simulink model "VirtualADAPT"  (Simulink 8.9)
settings: Solver=ode45  StartTime=0.0  StopTime=inf  RelTol=1e-3  EnableMultiTasking=on
PostLoadFcn:
  | FaultInjectionGUIHandle = FaultInjectionGUI;
InitFcn:
  | VirtualADAPTDataStructure
undefined symbols (not defined in the file or in its folder): ln
libraries: simulink x363
(part 1 of 26)
CloseFcn:
  | close(FaultInjectionGUIHandle);
settings Model: SuppressorTable=22 serialization::archive 11 0 6 0 0 0 0 0  Creator=Indranil Roychoudhury and Matthew Daigle  LastModifiedBy=iroychou
settings OptimizationCC: ExpressionDepthLimit=2147483647  InitFltsAndDblsToZero=on
settings DebuggingCC: ReadBeforeWriteMsg=DisableAll  WriteAfterWriteMsg=DisableAll  WriteAfterReadMsg=DisableAll  SaveWithParameterizedLinksMsg=none  SolverPrmCheckMsg=warning  InheritedTsInSrcMsg=none  UnconnectedInputMsg=none  UnconnectedOutputMsg=none  StrictBusMsg=Warning
settings GRTTargetCC: TargetFcnLib=ansi_tfl_tmw.mat

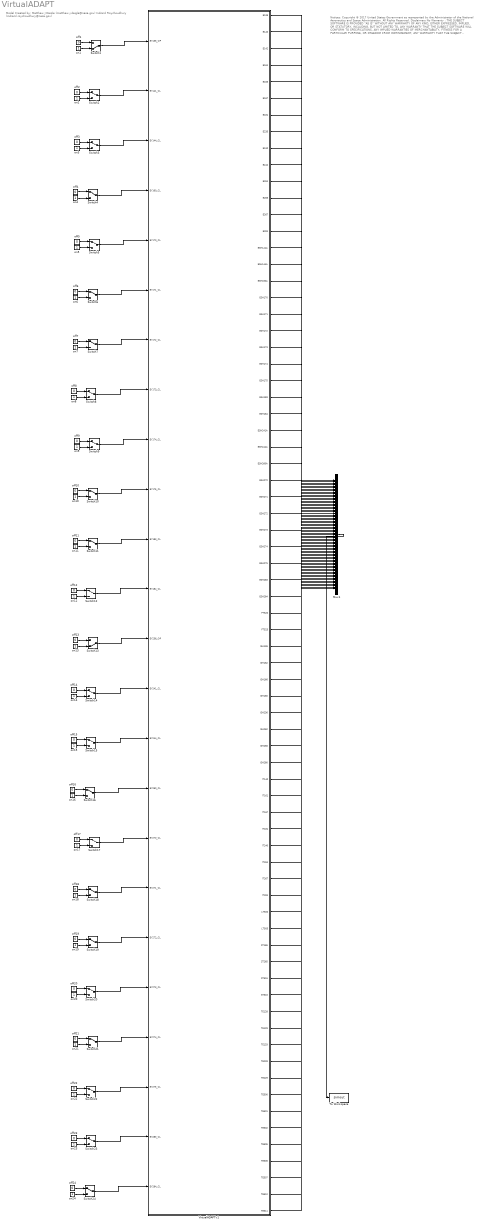
== system VirtualADAPT ==
  note: Notices:
      |
      | Copyright © 2017 United States Government as represented by the Administrator of the National Aeronautics and Space Administration. All Rights Reserved.
      |
      | Disclaimers
      |
      | No Warranty : THE SUBJECT SOFTWARE IS PROVIDED "AS IS" WITHOUT ANY WARRANTY OF ANY KIND, EITHER EXPRESSED, IMPLIED, OR STATUTORY, INCLUDING, BUT NOT LIMITED TO, ANY WARRANTY THAT THE SUBJECT SOFTWARE WILL CONFORM TO SPECIFICATIONS, ANY IMPLIED WARRANTIES OF MERCHANTABILITY, FITNESS FOR A PARTICULAR PURPOSE, OR FREEDOM FROM INFRINGEMENT, ANY WARRANTY THAT THE SUBJECT SOFTWARE WILL BE ERROR FREE, OR ANY WARRANTY THAT DOCUMENTATION, IF PROVIDED, WILL CONFORM TO THE SUBJECT SOFTWARE. THIS AGREEMENT DOES NOT, IN ANY MANNER, CONSTITUTE AN ENDORSEMENT BY GOVERNMENT AGENCY OR ANY PRIOR RECIPIENT OF ANY RESULTS, RESULTING DESIGNS, HARDWARE, SOFTWARE PRODUCTS OR ANY OTHER APPLICATIONS RESULTING FROM USE OF THE SUBJECT SOFTWARE. FURTHER, GOVERNMENT AGENCY DISCLAIMS ALL WARRANTIES AND LIABILITIES REGARDING THIRD-PARTY SOFTWARE, IF PRESENT IN THE ORIGINAL SOFTWARE, AND DISTRIBUTES IT "AS IS."
      |
      | Waiver and Indemnity: RECIPIENT AGREES TO WAIVE ANY AND ALL CLAIMS AGAINST THE UNITED STATES GOVERNMENT, ITS CONTRACTORS AND SUBCONTRACTORS, AS WELL AS ANY PRIOR RECIPIENT. IF RECIPIENT'S USE OF THE SUBJECT SOFTWARE RESULTS IN ANY LIABILITIES, DEMANDS, DAMAGES, EXPENSES OR LOSSES ARISING FROM SUCH USE, INCLUDING ANY DAMAGES FROM PRODUCTS BASED ON, OR RESULTING FROM, RECIPIENT'S USE OF THE SUBJECT SOFTWARE, RECIPIENT SHALL INDEMNIFY AND HOLD HARMLESS THE UNITED STATES GOVERNMENT, ITS CONTRACTORS AND SUBCONTRACTORS, AS WELL AS ANY PRIOR RECIPIENT, TO THE EXTENT PERMITTED BY LAW. RECIPIENT'S SOLE REMEDY FOR ANY SUCH MATTER SHALL BE THE IMMEDIATE, UNILATERAL TERMINATION OF THIS AGREEMENT.
  note: Model Created by:
      | Matthew J Daigle (matthew.j.daigle@nasa.gov)
      | Indranil Roychoudhury (indranil.roychoudhury@nasa.gov)
  Mux1 : Mux  Inputs=73
  Switch1 : ManualSwitch  CurrentSetting=0
  Switch{2..12,14..24} (22 alike) : ManualSwitch
  Switch13 : ManualSwitch  CurrentSetting=0
  To Workspace : ToWorkspace  VariableName=simout  SaveFormat=Structure  SampleTime=-1
  VirtualADAPTv1 : Subsystem
  off{1..24} (24 alike) : Constant  Value=0
  on{1..24} (24 alike) : Constant
  wires:
  off1/1 -> Switch1/1
  on1/1 -> Switch1/2
  off2/1 -> Switch2/1
  on2/1 -> Switch2/2
  off3/1 -> Switch3/1
  on3/1 -> Switch3/2
  off4/1 -> Switch4/1
  on4/1 -> Switch4/2
  off5/1 -> Switch5/1
  on5/1 -> Switch5/2
  off6/1 -> Switch6/1
  on6/1 -> Switch6/2
  off7/1 -> Switch7/1
  on7/1 -> Switch7/2
  off8/1 -> Switch8/1
  on8/1 -> Switch8/2
  off9/1 -> Switch9/1
  on9/1 -> Switch9/2
  off10/1 -> Switch10/1
  on10/1 -> Switch10/2
  off11/1 -> Switch11/1
  on11/1 -> Switch11/2
  off12/1 -> Switch12/1
  on12/1 -> Switch12/2
  off13/1 -> Switch13/1
  on13/1 -> Switch13/2
  off14/1 -> Switch14/1
  on14/1 -> Switch14/2
  off15/1 -> Switch15/1
  on15/1 -> Switch15/2
  off16/1 -> Switch16/1
  on16/1 -> Switch16/2
  off17/1 -> Switch17/1
  on17/1 -> Switch17/2
  off18/1 -> Switch18/1
  on18/1 -> Switch18/2
  off19/1 -> Switch19/1
  on19/1 -> Switch19/2
  off20/1 -> Switch20/1
  on20/1 -> Switch20/2
  off21/1 -> Switch21/1
  on21/1 -> Switch21/2
  off22/1 -> Switch22/1
  on22/1 -> Switch22/2
  off23/1 -> Switch23/1
  on23/1 -> Switch23/2
  off24/1 -> Switch24/1
  on24/1 -> Switch24/2
  Mux1/1 -> To Workspace/1
  Switch1/1 -> VirtualADAPTv1/1(EY136_OP)
  Switch2/1 -> VirtualADAPTv1/2(EY141_CL)
  Switch3/1 -> VirtualADAPTv1/3(EY144_CL)
  Switch4/1 -> VirtualADAPTv1/4(EY160_CL)
  Switch5/1 -> VirtualADAPTv1/5(EY170_CL)
  Switch6/1 -> VirtualADAPTv1/6(EY171_CL)
  Switch7/1 -> VirtualADAPTv1/7(EY172_CL)
  Switch8/1 -> VirtualADAPTv1/8(EY173_CL)
  Switch9/1 -> VirtualADAPTv1/9(EY174_CL)
  Switch10/1 -> VirtualADAPTv1/10(EY175_CL)
  Switch11/1 -> VirtualADAPTv1/11(EY183_CL)
  Switch12/1 -> VirtualADAPTv1/12(EY184_CL)
  Switch13/1 -> VirtualADAPTv1/13(EY236_OP)
  Switch14/1 -> VirtualADAPTv1/14(EY241_CL)
  Switch15/1 -> VirtualADAPTv1/15(EY244_CL)
  Switch16/1 -> VirtualADAPTv1/16(EY260_CL)
  Switch17/1 -> VirtualADAPTv1/17(EY270_CL)
  Switch18/1 -> VirtualADAPTv1/18(EY271_CL)
  Switch19/1 -> VirtualADAPTv1/19(EY272_CL)
  Switch20/1 -> VirtualADAPTv1/20(EY273_CL)
  Switch21/1 -> VirtualADAPTv1/21(EY274_CL)
  Switch22/1 -> VirtualADAPTv1/22(EY275_CL)
  Switch23/1 -> VirtualADAPTv1/23(EY283_CL)
  Switch24/1 -> VirtualADAPTv1/24(EY284_CL)
  VirtualADAPTv1/1(E135) -> Mux1/1
  VirtualADAPTv1/2(E140) -> Mux1/2
  VirtualADAPTv1/3(E142) -> Mux1/3
  VirtualADAPTv1/4(E161) -> Mux1/4
  VirtualADAPTv1/5(E165) -> Mux1/5
  VirtualADAPTv1/6(E167) -> Mux1/6
  VirtualADAPTv1/7(E181) -> Mux1/7
  VirtualADAPTv1/8(E235) -> Mux1/8
  VirtualADAPTv1/9(E240) -> Mux1/9
  VirtualADAPTv1/10(E242) -> Mux1/10
  VirtualADAPTv1/11(E261) -> Mux1/11
  VirtualADAPTv1/12(E265) -> Mux1/12
  VirtualADAPTv1/13(E267) -> Mux1/13
  VirtualADAPTv1/14(E281) -> Mux1/14
  VirtualADAPTv1/15(ESH141A) -> Mux1/15
  VirtualADAPTv1/16(ESH144A) -> Mux1/16
  VirtualADAPTv1/17(ESH160A) -> Mux1/17
  VirtualADAPTv1/18(ESH170) -> Mux1/18
  VirtualADAPTv1/19(ESH171) -> Mux1/19
  VirtualADAPTv1/20(ESH172) -> Mux1/20
  VirtualADAPTv1/21(ESH173) -> Mux1/21
  VirtualADAPTv1/22(ESH174) -> Mux1/22
  VirtualADAPTv1/23(ESH175) -> Mux1/23
  VirtualADAPTv1/24(ESH183) -> Mux1/24
  VirtualADAPTv1/25(ESH184) -> Mux1/25
  VirtualADAPTv1/26(ESH241A) -> Mux1/26
  VirtualADAPTv1/27(ESH244A) -> Mux1/27
  VirtualADAPTv1/28(ESH260A) -> Mux1/28
  VirtualADAPTv1/29(ESH270) -> Mux1/29
  VirtualADAPTv1/30(ESH271) -> Mux1/30
  VirtualADAPTv1/31(ESH272) -> Mux1/31
  VirtualADAPTv1/32(ESH273) -> Mux1/32
  VirtualADAPTv1/33(ESH274) -> Mux1/33
  VirtualADAPTv1/34(ESH275) -> Mux1/34
  VirtualADAPTv1/35(ESH283) -> Mux1/35
  VirtualADAPTv1/36(ESH284) -> Mux1/36
  VirtualADAPTv1/37(FT520) -> Mux1/37
  VirtualADAPTv1/38(FT525) -> Mux1/38
  VirtualADAPTv1/39(ISH136) -> Mux1/39
  VirtualADAPTv1/40(ISH162) -> Mux1/40
  VirtualADAPTv1/41(ISH166) -> Mux1/41
  VirtualADAPTv1/42(ISH180) -> Mux1/42
  VirtualADAPTv1/43(ISH236) -> Mux1/43
  VirtualADAPTv1/44(ISH262) -> Mux1/44
  VirtualADAPTv1/45(ISH266) -> Mux1/45
  VirtualADAPTv1/46(ISH280) -> Mux1/46
  VirtualADAPTv1/47(IT140) -> Mux1/47
  VirtualADAPTv1/48(IT161) -> Mux1/48
  VirtualADAPTv1/49(IT167) -> Mux1/49
  VirtualADAPTv1/50(IT181) -> Mux1/50
  VirtualADAPTv1/51(IT240) -> Mux1/51
  VirtualADAPTv1/52(IT261) -> Mux1/52
  VirtualADAPTv1/53(IT267) -> Mux1/53
  VirtualADAPTv1/54(IT281) -> Mux1/54
  VirtualADAPTv1/55(LT500) -> Mux1/55
  VirtualADAPTv1/56(LT505) -> Mux1/56
  VirtualADAPTv1/57(ST165) -> Mux1/57
  VirtualADAPTv1/58(ST265) -> Mux1/58
  VirtualADAPTv1/59(ST515) -> Mux1/59
  VirtualADAPTv1/60(ST516) -> Mux1/60
  VirtualADAPTv1/61(TE128) -> Mux1/61
  VirtualADAPTv1/62(TE129) -> Mux1/62
  VirtualADAPTv1/63(TE133) -> Mux1/63
  VirtualADAPTv1/64(TE228) -> Mux1/64
  VirtualADAPTv1/65(TE229) -> Mux1/65
  VirtualADAPTv1/66(TE500) -> Mux1/66
  VirtualADAPTv1/67(TE501) -> Mux1/67
  VirtualADAPTv1/68(TE502) -> Mux1/68
  VirtualADAPTv1/69(TE505) -> Mux1/69
  VirtualADAPTv1/70(TE506) -> Mux1/70
  VirtualADAPTv1/71(TE507) -> Mux1/71
  VirtualADAPTv1/72(TE510) -> Mux1/72
  VirtualADAPTv1/73(TE511) -> Mux1/73

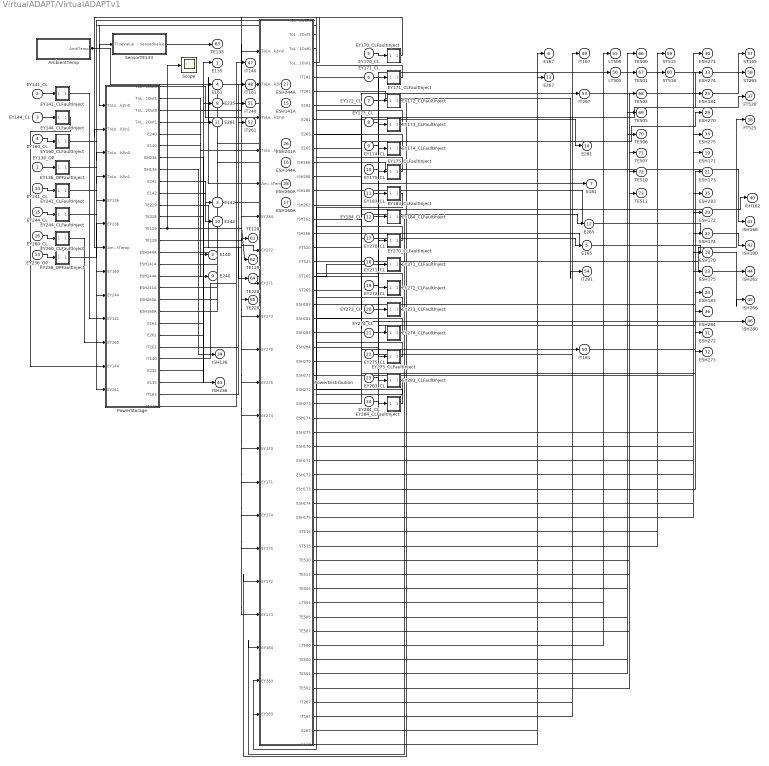
== system VirtualADAPT/VirtualADAPTv1 ==
  EY136_OP : Inport
  EY141_CL : Inport  Port=2
  EY144_CL : Inport  Port=3
  EY160_CL : Inport  Port=4
  EY170_CL : Inport  Port=5
  EY171_CL : Inport  Port=6
  EY172_CL : Inport  Port=7
  EY173_CL : Inport  Port=8
  EY174_CL : Inport  Port=9
  EY175_CL : Inport  Port=10
  EY183_CL : Inport  Port=11
  EY184_CL : Inport  Port=12
  EY236_OP : Inport  Port=13
  EY241_CL : Inport  Port=14
  EY244_CL : Inport  Port=15
  EY260_CL : Inport  Port=16
  EY270_CL : Inport  Port=17
  EY271_CL : Inport  Port=18
  EY272_CL : Inport  Port=19
  EY273_CL : Inport  Port=20
  EY274_CL : Inport  Port=21
  EY275_CL : Inport  Port=22
  EY283_CL : Inport  Port=23
  EY284_CL : Inport  Port=24
  AmbientTemp : Subsystem
  EY136_OPFaultInject : Subsystem  mask{FP=Nominal, FT=0, FM=1}
      mask prompts: FP "Fault Profile:"; FT "Fault Injection Time:"; FM "Stuck Value"
      mask TypeOptions FP: Nominal | StuckAt
      mask Display: image(imread('FaultInject.png','BackgroundColor',[1 1 1]))
  EY141_CLFaultInject : Subsystem  mask{FP=Nominal, FT=0, FM=0}
      mask prompts: FP "Fault Profile:"; FT "Fault Injection Time:"; FM "Stuck Value"
      mask TypeOptions FP: Nominal | StuckAt
      mask Display: image(imread('FaultInject.png','BackgroundColor',[1 1 1]))
  EY144_CLFaultInject : Subsystem  mask{FP=Nominal, FT=0, FM=0}
      mask prompts: FP "Fault Profile:"; FT "Fault Injection Time:"; FM "Stuck Value"
      mask TypeOptions FP: Nominal | StuckAt
      mask Display: image(imread('FaultInject.png','BackgroundColor',[1 1 1]))
  EY160_CLFaultInject : Subsystem  mask{FP=Nominal, FT=0, FM=0}
      mask prompts: FP "Fault Profile:"; FT "Fault Injection Time:"; FM "Stuck Value"
      mask TypeOptions FP: Nominal | StuckAt
      mask Display: image(imread('FaultInject.png','BackgroundColor',[1 1 1]))
  EY170_CLFaultInject : Subsystem  mask{FP=Nominal, FT=0, FM=0}
      mask prompts: FP "Fault Profile:"; FT "Fault Injection Time:"; FM "Stuck Value"
      mask TypeOptions FP: Nominal | StuckAt
      mask Display: image(imread('FaultInject.png','BackgroundColor',[1 1 1]))
  EY171_CLFaultInject : Subsystem  mask{FP=Nominal, FT=0, FM=0}
      mask prompts: FP "Fault Profile:"; FT "Fault Injection Time:"; FM "Stuck Value"
      mask TypeOptions FP: Nominal | StuckAt
      mask Display: image(imread('FaultInject.png','BackgroundColor',[1 1 1]))
  EY172_CLFaultInject : Subsystem  mask{FP=Nominal, FT=0, FM=0}
      mask prompts: FP "Fault Profile:"; FT "Fault Injection Time:"; FM "Stuck Value"
      mask TypeOptions FP: Nominal | StuckAt
      mask Display: image(imread('FaultInject.png','BackgroundColor',[1 1 1]))
  EY173_CLFaultInject : Subsystem  mask{FP=Nominal, FT=0, FM=0}
      mask prompts: FP "Fault Profile:"; FT "Fault Injection Time:"; FM "Stuck Value"
      mask TypeOptions FP: Nominal | StuckAt
      mask Display: image(imread('FaultInject.png','BackgroundColor',[1 1 1]))
  EY174_CLFaultInject : Subsystem  mask{FP=Nominal, FT=0, FM=0}
      mask prompts: FP "Fault Profile:"; FT "Fault Injection Time:"; FM "Stuck Value"
      mask TypeOptions FP: Nominal | StuckAt
      mask Display: image(imread('FaultInject.png','BackgroundColor',[1 1 1]))
  EY175_CLFaultInject : Subsystem  mask{FP=Nominal, FT=0, FM=0}
      mask prompts: FP "Fault Profile:"; FT "Fault Injection Time:"; FM "Stuck Value"
      mask TypeOptions FP: Nominal | StuckAt
      mask Display: image(imread('FaultInject.png','BackgroundColor',[1 1 1]))
  EY183_CLFaultInject : Subsystem  mask{FP=Nominal, FT=0, FM=0}
      mask prompts: FP "Fault Profile:"; FT "Fault Injection Time:"; FM "Stuck Value"
      mask TypeOptions FP: Nominal | StuckAt
      mask Display: image(imread('FaultInject.png','BackgroundColor',[1 1 1]))
  EY184_CLFaultInject : Subsystem  mask{FP=Nominal, FT=0, FM=0}
      mask prompts: FP "Fault Profile:"; FT "Fault Injection Time:"; FM "Stuck Value"
      mask TypeOptions FP: Nominal | StuckAt
      mask Display: image(imread('FaultInject.png','BackgroundColor',[1 1 1]))
  EY236_OPFaultInject : Subsystem  mask{FP=Nominal, FT=0, FM=0}
      mask prompts: FP "Fault Profile:"; FT "Fault Injection Time:"; FM "Stuck Value"
      mask TypeOptions FP: Nominal | StuckAt
      mask Display: image(imread('FaultInject.png','BackgroundColor',[1 1 1]))
  EY241_CLFaultInject : Subsystem  mask{FP=Nominal, FT=0, FM=0}
      mask prompts: FP "Fault Profile:"; FT "Fault Injection Time:"; FM "Stuck Value"
      mask TypeOptions FP: Nominal | StuckAt
      mask Display: image(imread('FaultInject.png','BackgroundColor',[1 1 1]))
  EY244_CLFaultInject : Subsystem  mask{FP=Nominal, FT=0, FM=0}
      mask prompts: FP "Fault Profile:"; FT "Fault Injection Time:"; FM "Stuck Value"
      mask TypeOptions FP: Nominal | StuckAt
      mask Display: image(imread('FaultInject.png','BackgroundColor',[1 1 1]))
  EY260_CLFaultInject : Subsystem  mask{FP=Nominal, FT=0, FM=0}
      mask prompts: FP "Fault Profile:"; FT "Fault Injection Time:"; FM "Stuck Value"
      mask TypeOptions FP: Nominal | StuckAt
      mask Display: image(imread('FaultInject.png','BackgroundColor',[1 1 1]))
  EY270_CLFaultInject : Subsystem  mask{FP=Nominal, FT=0, FM=0}
      mask prompts: FP "Fault Profile:"; FT "Fault Injection Time:"; FM "Stuck Value"
      mask TypeOptions FP: Nominal | StuckAt
      mask Display: image(imread('FaultInject.png','BackgroundColor',[1 1 1]))
  EY271_CLFaultInject : Subsystem  mask{FP=Nominal, FT=0, FM=0}
      mask prompts: FP "Fault Profile:"; FT "Fault Injection Time:"; FM "Stuck Value"
      mask TypeOptions FP: Nominal | StuckAt
      mask Display: image(imread('FaultInject.png','BackgroundColor',[1 1 1]))
  EY272_CLFaultInject : Subsystem  mask{FP=Nominal, FT=0, FM=0}
      mask prompts: FP "Fault Profile:"; FT "Fault Injection Time:"; FM "Stuck Value"
      mask TypeOptions FP: Nominal | StuckAt
      mask Display: image(imread('FaultInject.png','BackgroundColor',[1 1 1]))
  EY273_CLFaultInject : Subsystem  mask{FP=Nominal, FT=0, FM=0}
      mask prompts: FP "Fault Profile:"; FT "Fault Injection Time:"; FM "Stuck Value"
      mask TypeOptions FP: Nominal | StuckAt
      mask Display: image(imread('FaultInject.png','BackgroundColor',[1 1 1]))
  EY274_CLFaultInject : Subsystem  mask{FP=Nominal, FT=0, FM=0}
      mask prompts: FP "Fault Profile:"; FT "Fault Injection Time:"; FM "Stuck Value"
      mask TypeOptions FP: Nominal | StuckAt
      mask Display: image(imread('FaultInject.png','BackgroundColor',[1 1 1]))
  EY275_CLFaultInject : Subsystem  mask{FP=Nominal, FT=0, FM=0}
      mask prompts: FP "Fault Profile:"; FT "Fault Injection Time:"; FM "Stuck Value"
      mask TypeOptions FP: Nominal | StuckAt
      mask Display: image(imread('FaultInject.png','BackgroundColor',[1 1 1]))
  EY283_CLFaultInject : Subsystem  mask{FP=Nominal, FT=0, FM=0}
      mask prompts: FP "Fault Profile:"; FT "Fault Injection Time:"; FM "Stuck Value"
      mask TypeOptions FP: Nominal | StuckAt
      mask Display: image(imread('FaultInject.png','BackgroundColor',[1 1 1]))
  EY284_CLFaultInject : Subsystem  mask{FP=Nominal, FT=0, FM=0}
      mask prompts: FP "Fault Profile:"; FT "Fault Injection Time:"; FM "Stuck Value"
      mask TypeOptions FP: Nominal | StuckAt
      mask Display: image(imread('FaultInject.png','BackgroundColor',[1 1 1]))
  PowerDistribution : Subsystem
  PowerStorage : Subsystem
  Scope : Scope
  SensorTE133 : Subsystem
  E135 : Outport
  E140 : Outport  Port=2
  E142 : Outport  Port=3
  E161 : Outport  Port=4
  E165 : Outport  Port=5
  E167 : Outport  Port=6
  E181 : Outport  Port=7
  E235 : Outport  Port=8
  E240 : Outport  Port=9
  E242 : Outport  Port=10
  E261 : Outport  Port=11
  E265 : Outport  Port=12
  E267 : Outport  Port=13
  E281 : Outport  Port=14
  ESH141A : Outport  Port=15
  ESH144A : Outport  Port=16
  ESH160A : Outport  Port=17
  ESH170 : Outport  Port=18
  ESH171 : Outport  Port=19
  ESH172 : Outport  Port=20
  ESH173 : Outport  Port=21
  ESH174 : Outport  Port=22
  ESH175 : Outport  Port=23
  ESH183 : Outport  Port=24
  ESH184 : Outport  Port=25
  ESH241A : Outport  Port=26
  ESH244A : Outport  Port=27
  ESH260A : Outport  Port=28
  ESH270 : Outport  Port=29
  ESH271 : Outport  Port=30
  ESH272 : Outport  Port=31
  ESH273 : Outport  Port=32
  ESH274 : Outport  Port=33
  ESH275 : Outport  Port=34
  ESH283 : Outport  Port=35
  ESH284 : Outport  Port=36
  FT520 : Outport  Port=37
  FT525 : Outport  Port=38
  ISH136 : Outport  Port=39
  ISH162 : Outport  Port=40
  ISH166 : Outport  Port=41
  ISH180 : Outport  Port=42
  ISH236 : Outport  Port=43
  ISH262 : Outport  Port=44
  ISH266 : Outport  Port=45
  ISH280 : Outport  Port=46
  IT140 : Outport  Port=47
  IT161 : Outport  Port=48
  IT167 : Outport  Port=49
  IT181 : Outport  Port=50
  IT240 : Outport  Port=51
  IT261 : Outport  Port=52
  IT267 : Outport  Port=53
  IT281 : Outport  Port=54
  LT500 : Outport  Port=55
  LT505 : Outport  Port=56
  ST165 : Outport  Port=57
  ST265 : Outport  Port=58
  ST515 : Outport  Port=59
  ST516 : Outport  Port=60
  TE128 : Outport  Port=61
  TE129 : Outport  Port=62
  TE133 : Outport  Port=63
  TE228 : Outport  Port=64
  TE229 : Outport  Port=65
  TE500 : Outport  Port=66
  TE501 : Outport  Port=67
  TE502 : Outport  Port=68
  TE505 : Outport  Port=69
  TE506 : Outport  Port=70
  TE507 : Outport  Port=71
  TE510 : Outport  Port=72
  TE511 : Outport  Port=73
  wires:
  EY271_CL/1 -> EY271_CLFaultInject/1(In)
  EY270_CL/1 -> EY270_CLFaultInject/1(In)
  EY275_CL/1 -> EY275_CLFaultInject/1(In)
  EY174_CL/1 -> EY174_CLFaultInject/1(In)
  EY170_CL/1 -> EY170_CLFaultInject/1(In)
  EY273_CL/1 -> EY273_CLFaultInject/1(In)
  EY175_CL/1 -> EY175_CLFaultInject/1(In)
  EY171_CL/1 -> EY171_CLFaultInject/1(In)
  EY172_CL/1 -> EY172_CLFaultInject/1(In)
  EY272_CL/1 -> EY272_CLFaultInject/1(In)
  EY283_CL/1 -> EY283_CLFaultInject/1(In)
  EY173_CL/1 -> EY173_CLFaultInject/1(In)
  EY184_CL/1 -> EY184_CLFaultInject/1(In)
  EY183_CL/1 -> EY183_CLFaultInject/1(In)
  EY260_CL/1 -> EY260_CLFaultInject/1(In)
  EY141_CL/1 -> EY141_CLFaultInject/1(In)
  EY144_CL/1 -> EY144_CLFaultInject/1(In)
  EY160_CL/1 -> EY160_CLFaultInject/1(In)
  EY244_CL/1 -> EY244_CLFaultInject/1(In)
  EY241_CL/1 -> EY241_CLFaultInject/1(In)
  EY136_OP/1 -> EY136_OPFaultInject/1(In)
  EY284_CL/1 -> EY284_CLFaultInject/1(In)
  EY274_CL/1 -> EY274_CLFaultInject/1(In)
  EY236_OP/1 -> EY236_OPFaultInject/1(In)
  PowerStorage/1(ToLoadBank1Out0) -> PowerDistribution/3(ToLoadBank1In0)
  PowerDistribution/3(ToLoadBank1Out0) -> PowerStorage/1(ToLoadBank1In0)
  PowerStorage/2(ToLoadBank1Out1) -> PowerDistribution/4(ToLoadBank1In1)
  PowerDistribution/4(ToLoadBank1Out1) -> PowerStorage/2(ToLoadBank1In1)
  PowerStorage/3(ToLoadBank2Out0) -> PowerDistribution/1(ToLoadBank2In0)
  PowerDistribution/1(ToLoadBank2Out0) -> PowerStorage/3(ToLoadBank2In0)
  PowerStorage/4(ToLoadBank2Out1) -> PowerDistribution/2(ToLoadBank2In1)
  PowerDistribution/2(ToLoadBank2Out1) -> PowerStorage/4(ToLoadBank2In1)
  AmbientTemp/1(AmbTemp) -> PowerDistribution/5(AmbientTemp), SensorTE133/1(TrueValue), PowerStorage/7(AmbientTemp)
  EY283_CLFaultInject/1(Out) -> PowerDistribution/20(EY283)
  EY184_CLFaultInject/1(Out) -> PowerDistribution/19(EY184)
  EY170_CLFaultInject/1(Out) -> PowerDistribution/13(EY170)
  EY260_CLFaultInject/1(Out) -> PowerStorage/11(EY260)
  EY273_CLFaultInject/1(Out) -> PowerDistribution/9(EY273)
  EY271_CLFaultInject/1(Out) -> PowerDistribution/8(EY271)
  EY144_CLFaultInject/1(Out) -> PowerStorage/12(EY144)
  EY141_CLFaultInject/1(Out) -> PowerStorage/10(EY141)
  EY136_OPFaultInject/1(Out) -> PowerStorage/5(EY136)
  EY275_CLFaultInject/1(Out) -> PowerDistribution/11(EY275)
  EY284_CLFaultInject/1(Out) -> PowerDistribution/6(EY284)
  EY160_CLFaultInject/1(Out) -> PowerStorage/8(EY160)
  EY244_CLFaultInject/1(Out) -> PowerStorage/9(EY244)
  EY236_OPFaultInject/1(Out) -> PowerStorage/6(EY236)
  EY241_CLFaultInject/1(Out) -> PowerStorage/13(EY241)
  EY172_CLFaultInject/1(Out) -> PowerDistribution/17(EY172)
  EY270_CLFaultInject/1(Out) -> PowerDistribution/10(EY270)
  EY274_CLFaultInject/1(Out) -> PowerDistribution/12(EY274)
  EY175_CLFaultInject/1(Out) -> PowerDistribution/16(EY175)
  EY174_CLFaultInject/1(Out) -> PowerDistribution/15(EY174)
  EY173_CLFaultInject/1(Out) -> PowerDistribution/18(EY173)
  EY272_CLFaultInject/1(Out) -> PowerDistribution/7(EY272)
  EY183_CLFaultInject/1(Out) -> PowerDistribution/21(EY183)
  EY171_CLFaultInject/1(Out) -> PowerDistribution/14(EY171)
  PowerDistribution/34(ESH173) -> ESH173/1
  PowerDistribution/27(ESH272) -> ESH272/1
  PowerDistribution/35(ESH174) -> ESH174/1
  PowerDistribution/36(ESH175) -> ESH175/1
  PowerDistribution/24(ESH284) -> ESH284/1
  PowerStorage/21(E161) -> E161/1
  PowerStorage/26(E135) -> E135/1
  PowerStorage/25(E235) -> E235/1
  PowerStorage/22(E261) -> E261/1
  PowerDistribution/11(ISH166) -> ISH166/1
  PowerStorage/27(IT161) -> IT161/1
  PowerStorage/23(IT261) -> IT261/1
  PowerDistribution/48(TE502) -> TE502/1
  PowerDistribution/14(ISH262) -> ISH262/1
  PowerDistribution/45(LT500) -> LT500/1
  PowerStorage/11(TE229) -> TE229/1
  PowerDistribution/13(ISH180) -> ISH180/1
  PowerDistribution/12(ISH280) -> ISH280/1
  PowerDistribution/16(ISH266) -> ISH266/1
  PowerDistribution/10(E165) -> E165/1
  PowerDistribution/50(IT167) -> IT167/1
  PowerDistribution/25(ESH270) -> ESH270/1
  PowerDistribution/17(FT520) -> FT520/1
  PowerDistribution/20(ST265) -> ST265/1
  PowerStorage/16(ESH141A) -> ESH141A/1
  PowerDistribution/30(ESH275) -> ESH275/1
  PowerDistribution/15(ISH162) -> ISH162/1
  PowerDistribution/52(E167) -> E167/1
  PowerDistribution/42(LT505) -> LT505/1
  SensorTE133/1(SensedValue) -> TE133/1
  PowerDistribution/8(E281) -> E281/1
  PowerStorage/18(ESH241A) -> ESH241A/1
  PowerStorage/7(ISH236) -> ISH236/1
  PowerDistribution/51(E267) -> E267/1
  PowerStorage/15(ESH144A) -> ESH144A/1
  PowerStorage/5(E240) -> E240/1
  PowerDistribution/47(TE501) -> TE501/1
  PowerDistribution/22(ESH184) -> ESH184/1
  PowerDistribution/18(FT525) -> FT525/1
  PowerDistribution/38(ST515) -> ST515/1
  PowerDistribution/28(ESH273) -> ESH273/1
  PowerDistribution/21(ESH183) -> ESH183/1
  PowerDistribution/44(TE507) -> TE507/1
  PowerDistribution/46(TE500) -> TE500/1
  PowerDistribution/29(ESH274) -> ESH274/1
  PowerDistribution/23(ESH283) -> ESH283/1
  PowerDistribution/39(TE510) -> TE510/1
  PowerDistribution/32(ESH171) -> ESH171/1
  PowerStorage/17(ESH244A) -> ESH244A/1
  PowerDistribution/41(TE505) -> TE505/1
  PowerStorage/13(TE129) -> TE129/1, Scope/1
  PowerStorage/14(TE128) -> TE128/1
  PowerDistribution/26(ESH271) -> ESH271/1
  PowerDistribution/33(ESH172) -> ESH172/1
  PowerDistribution/7(E181) -> E181/1
  PowerDistribution/43(TE506) -> TE506/1
  PowerStorage/20(ESH160A) -> ESH160A/1
  PowerStorage/6(E140) -> E140/1
  PowerStorage/9(E242) -> E242/1
  PowerStorage/12(TE228) -> TE228/1
  PowerStorage/10(E142) -> E142/1
  PowerStorage/19(ESH260A) -> ESH260A/1
  PowerStorage/24(IT140) -> IT140/1
  PowerDistribution/9(E265) -> E265/1
  PowerDistribution/49(IT267) -> IT267/1
  PowerDistribution/6(IT281) -> IT281/1
  PowerDistribution/40(TE511) -> TE511/1
  PowerStorage/8(ISH136) -> ISH136/1
  PowerStorage/28(IT240) -> IT240/1
  PowerDistribution/37(ST516) -> ST516/1
  PowerDistribution/31(ESH170) -> ESH170/1
  PowerDistribution/19(ST165) -> ST165/1
  PowerDistribution/5(IT181) -> IT181/1

== system VirtualADAPT/VirtualADAPTv1/AmbientTemp ==
  AmbientTemp : Subsystem  mask{Constant=22, FP=Nominal, FT=0, FM=0}
      mask prompts: Constant "Value of Constant:"; FP "Fault Profile:"; FT "Fault Injection Time:"; FM "Fault Magnitude/Slope"
      mask TypeOptions FP: Nominal | Abrupt | Incipient
      mask Display: image(imread('Constant.png','BackgroundColor',[1 1 1]))
  AmbTemp : Outport
  wires:
  AmbientTemp/1(C) -> AmbTemp/1

== system VirtualADAPT/VirtualADAPTv1/EY136_OPFaultInject ==
  In : Inport
  Fault Inject : Subsystem
  Fault Profile : Step  Time=FT  Before=1  After=FP+(FP-1)*3  SampleTime=0
  Filler : Constant  Value=0
  StuckAt : Constant  Value=FM
  Out : Outport
  wires:
  Fault Profile/1 -> Fault Inject/2(Fault Profile)
  Filler/1 -> Fault Inject/3(Step), Fault Inject/4(Ramp), Fault Inject/5(Bias)
  In/1 -> Fault Inject/1(Nominal)
  Fault Inject/1(Out1) -> Out/1
  StuckAt/1 -> Fault Inject/6(stuckAt)

== system VirtualADAPT/VirtualADAPTv1/EY141_CLFaultInject ==
  In : Inport
  Fault Inject : Subsystem
  Fault Profile : Step  Time=FT  Before=1  After=FP+(FP-1)*3  SampleTime=0
  Filler : Constant  Value=0
  StuckAt : Constant  Value=FM
  Out : Outport
  wires:
  Fault Profile/1 -> Fault Inject/2(Fault Profile)
  Filler/1 -> Fault Inject/3(Step), Fault Inject/4(Ramp), Fault Inject/5(Bias)
  In/1 -> Fault Inject/1(Nominal)
  Fault Inject/1(Out1) -> Out/1
  StuckAt/1 -> Fault Inject/6(stuckAt)

== system VirtualADAPT/VirtualADAPTv1/EY144_CLFaultInject ==
  In : Inport
  Fault Inject : Subsystem
  Fault Profile : Step  Time=FT  Before=1  After=FP+(FP-1)*3  SampleTime=0
  Filler : Constant  Value=0
  StuckAt : Constant  Value=FM
  Out : Outport
  wires:
  Fault Profile/1 -> Fault Inject/2(Fault Profile)
  Filler/1 -> Fault Inject/3(Step), Fault Inject/4(Ramp), Fault Inject/5(Bias)
  In/1 -> Fault Inject/1(Nominal)
  Fault Inject/1(Out1) -> Out/1
  StuckAt/1 -> Fault Inject/6(stuckAt)

== system VirtualADAPT/VirtualADAPTv1/EY160_CLFaultInject ==
  In : Inport
  Fault Inject : Subsystem
  Fault Profile : Step  Time=FT  Before=1  After=FP+(FP-1)*3  SampleTime=0
  Filler : Constant  Value=0
  StuckAt : Constant  Value=FM
  Out : Outport
  wires:
  Fault Profile/1 -> Fault Inject/2(Fault Profile)
  Filler/1 -> Fault Inject/3(Step), Fault Inject/4(Ramp), Fault Inject/5(Bias)
  In/1 -> Fault Inject/1(Nominal)
  Fault Inject/1(Out1) -> Out/1
  StuckAt/1 -> Fault Inject/6(stuckAt)

== system VirtualADAPT/VirtualADAPTv1/EY170_CLFaultInject ==
  In : Inport
  Fault Inject : Subsystem
  Fault Profile : Step  Time=FT  Before=1  After=FP+(FP-1)*3  SampleTime=0
  Filler : Constant  Value=0
  StuckAt : Constant  Value=FM
  Out : Outport
  wires:
  Fault Profile/1 -> Fault Inject/2(Fault Profile)
  Filler/1 -> Fault Inject/3(Step), Fault Inject/4(Ramp), Fault Inject/5(Bias)
  In/1 -> Fault Inject/1(Nominal)
  Fault Inject/1(Out1) -> Out/1
  StuckAt/1 -> Fault Inject/6(stuckAt)

== system VirtualADAPT/VirtualADAPTv1/EY171_CLFaultInject ==
  In : Inport
  Fault Inject : Subsystem
  Fault Profile : Step  Time=FT  Before=1  After=FP+(FP-1)*3  SampleTime=0
  Filler : Constant  Value=0
  StuckAt : Constant  Value=FM
  Out : Outport
  wires:
  Fault Profile/1 -> Fault Inject/2(Fault Profile)
  Filler/1 -> Fault Inject/3(Step), Fault Inject/4(Ramp), Fault Inject/5(Bias)
  In/1 -> Fault Inject/1(Nominal)
  Fault Inject/1(Out1) -> Out/1
  StuckAt/1 -> Fault Inject/6(stuckAt)

== system VirtualADAPT/VirtualADAPTv1/EY172_CLFaultInject ==
  In : Inport
  Fault Inject : Subsystem
  Fault Profile : Step  Time=FT  Before=1  After=FP+(FP-1)*3  SampleTime=0
  Filler : Constant  Value=0
  StuckAt : Constant  Value=FM
  Out : Outport
  wires:
  Fault Profile/1 -> Fault Inject/2(Fault Profile)
  Filler/1 -> Fault Inject/3(Step), Fault Inject/4(Ramp), Fault Inject/5(Bias)
  In/1 -> Fault Inject/1(Nominal)
  Fault Inject/1(Out1) -> Out/1
  StuckAt/1 -> Fault Inject/6(stuckAt)

== system VirtualADAPT/VirtualADAPTv1/EY173_CLFaultInject ==
  In : Inport
  Fault Inject : Subsystem
  Fault Profile : Step  Time=FT  Before=1  After=FP+(FP-1)*3  SampleTime=0
  Filler : Constant  Value=0
  StuckAt : Constant  Value=FM
  Out : Outport
  wires:
  Fault Profile/1 -> Fault Inject/2(Fault Profile)
  Filler/1 -> Fault Inject/3(Step), Fault Inject/4(Ramp), Fault Inject/5(Bias)
  In/1 -> Fault Inject/1(Nominal)
  Fault Inject/1(Out1) -> Out/1
  StuckAt/1 -> Fault Inject/6(stuckAt)

== system VirtualADAPT/VirtualADAPTv1/EY174_CLFaultInject ==
  In : Inport
  Fault Inject : Subsystem
  Fault Profile : Step  Time=FT  Before=1  After=FP+(FP-1)*3  SampleTime=0
  Filler : Constant  Value=0
  StuckAt : Constant  Value=FM
  Out : Outport
  wires:
  Fault Profile/1 -> Fault Inject/2(Fault Profile)
  Filler/1 -> Fault Inject/3(Step), Fault Inject/4(Ramp), Fault Inject/5(Bias)
  In/1 -> Fault Inject/1(Nominal)
  Fault Inject/1(Out1) -> Out/1
  StuckAt/1 -> Fault Inject/6(stuckAt)

== system VirtualADAPT/VirtualADAPTv1/EY175_CLFaultInject ==
  In : Inport
  Fault Inject : Subsystem
  Fault Profile : Step  Time=FT  Before=1  After=FP+(FP-1)*3  SampleTime=0
  Filler : Constant  Value=0
  StuckAt : Constant  Value=FM
  Out : Outport
  wires:
  Fault Profile/1 -> Fault Inject/2(Fault Profile)
  Filler/1 -> Fault Inject/3(Step), Fault Inject/4(Ramp), Fault Inject/5(Bias)
  In/1 -> Fault Inject/1(Nominal)
  Fault Inject/1(Out1) -> Out/1
  StuckAt/1 -> Fault Inject/6(stuckAt)

== system VirtualADAPT/VirtualADAPTv1/EY183_CLFaultInject ==
  In : Inport
  Fault Inject : Subsystem
  Fault Profile : Step  Time=FT  Before=1  After=FP+(FP-1)*3  SampleTime=0
  Filler : Constant  Value=0
  StuckAt : Constant  Value=FM
  Out : Outport
  wires:
  Fault Profile/1 -> Fault Inject/2(Fault Profile)
  Filler/1 -> Fault Inject/3(Step), Fault Inject/4(Ramp), Fault Inject/5(Bias)
  In/1 -> Fault Inject/1(Nominal)
  Fault Inject/1(Out1) -> Out/1
  StuckAt/1 -> Fault Inject/6(stuckAt)

== system VirtualADAPT/VirtualADAPTv1/EY184_CLFaultInject ==
  In : Inport
  Fault Inject : Subsystem
  Fault Profile : Step  Time=FT  Before=1  After=FP+(FP-1)*3  SampleTime=0
  Filler : Constant  Value=0
  StuckAt : Constant  Value=FM
  Out : Outport
  wires:
  Fault Profile/1 -> Fault Inject/2(Fault Profile)
  Filler/1 -> Fault Inject/3(Step), Fault Inject/4(Ramp), Fault Inject/5(Bias)
  In/1 -> Fault Inject/1(Nominal)
  Fault Inject/1(Out1) -> Out/1
  StuckAt/1 -> Fault Inject/6(stuckAt)

== system VirtualADAPT/VirtualADAPTv1/EY236_OPFaultInject ==
  In : Inport
  Fault Inject : Subsystem
  Fault Profile : Step  Time=FT  Before=1  After=FP+(FP-1)*3  SampleTime=0
  Filler : Constant  Value=0
  StuckAt : Constant  Value=FM
  Out : Outport
  wires:
  Fault Profile/1 -> Fault Inject/2(Fault Profile)
  Filler/1 -> Fault Inject/3(Step), Fault Inject/4(Ramp), Fault Inject/5(Bias)
  In/1 -> Fault Inject/1(Nominal)
  Fault Inject/1(Out1) -> Out/1
  StuckAt/1 -> Fault Inject/6(stuckAt)

== system VirtualADAPT/VirtualADAPTv1/EY241_CLFaultInject ==
  In : Inport
  Fault Inject : Subsystem
  Fault Profile : Step  Time=FT  Before=1  After=FP+(FP-1)*3  SampleTime=0
  Filler : Constant  Value=0
  StuckAt : Constant  Value=FM
  Out : Outport
  wires:
  Fault Profile/1 -> Fault Inject/2(Fault Profile)
  Filler/1 -> Fault Inject/3(Step), Fault Inject/4(Ramp), Fault Inject/5(Bias)
  In/1 -> Fault Inject/1(Nominal)
  Fault Inject/1(Out1) -> Out/1
  StuckAt/1 -> Fault Inject/6(stuckAt)

== system VirtualADAPT/VirtualADAPTv1/EY244_CLFaultInject ==
  In : Inport
  Fault Inject : Subsystem
  Fault Profile : Step  Time=FT  Before=1  After=FP+(FP-1)*3  SampleTime=0
  Filler : Constant  Value=0
  StuckAt : Constant  Value=FM
  Out : Outport
  wires:
  Fault Profile/1 -> Fault Inject/2(Fault Profile)
  Filler/1 -> Fault Inject/3(Step), Fault Inject/4(Ramp), Fault Inject/5(Bias)
  In/1 -> Fault Inject/1(Nominal)
  Fault Inject/1(Out1) -> Out/1
  StuckAt/1 -> Fault Inject/6(stuckAt)

== system VirtualADAPT/VirtualADAPTv1/EY260_CLFaultInject ==
  In : Inport
  Fault Inject : Subsystem
  Fault Profile : Step  Time=FT  Before=1  After=FP+(FP-1)*3  SampleTime=0
  Filler : Constant  Value=0
  StuckAt : Constant  Value=FM
  Out : Outport
  wires:
  Fault Profile/1 -> Fault Inject/2(Fault Profile)
  Filler/1 -> Fault Inject/3(Step), Fault Inject/4(Ramp), Fault Inject/5(Bias)
  In/1 -> Fault Inject/1(Nominal)
  Fault Inject/1(Out1) -> Out/1
  StuckAt/1 -> Fault Inject/6(stuckAt)

== system VirtualADAPT/VirtualADAPTv1/EY270_CLFaultInject ==
  In : Inport
  Fault Inject : Subsystem
  Fault Profile : Step  Time=FT  Before=1  After=FP+(FP-1)*3  SampleTime=0
  Filler : Constant  Value=0
  StuckAt : Constant  Value=FM
  Out : Outport
  wires:
  Fault Profile/1 -> Fault Inject/2(Fault Profile)
  Filler/1 -> Fault Inject/3(Step), Fault Inject/4(Ramp), Fault Inject/5(Bias)
  In/1 -> Fault Inject/1(Nominal)
  Fault Inject/1(Out1) -> Out/1
  StuckAt/1 -> Fault Inject/6(stuckAt)

== system VirtualADAPT/VirtualADAPTv1/EY271_CLFaultInject ==
  In : Inport
  Fault Inject : Subsystem
  Fault Profile : Step  Time=FT  Before=1  After=FP+(FP-1)*3  SampleTime=0
  Filler : Constant  Value=0
  StuckAt : Constant  Value=FM
  Out : Outport
  wires:
  Fault Profile/1 -> Fault Inject/2(Fault Profile)
  Filler/1 -> Fault Inject/3(Step), Fault Inject/4(Ramp), Fault Inject/5(Bias)
  In/1 -> Fault Inject/1(Nominal)
  Fault Inject/1(Out1) -> Out/1
  StuckAt/1 -> Fault Inject/6(stuckAt)

== system VirtualADAPT/VirtualADAPTv1/EY272_CLFaultInject ==
  In : Inport
  Fault Inject : Subsystem
  Fault Profile : Step  Time=FT  Before=1  After=FP+(FP-1)*3  SampleTime=0
  Filler : Constant  Value=0
  StuckAt : Constant  Value=FM
  Out : Outport
  wires:
  Fault Profile/1 -> Fault Inject/2(Fault Profile)
  Filler/1 -> Fault Inject/3(Step), Fault Inject/4(Ramp), Fault Inject/5(Bias)
  In/1 -> Fault Inject/1(Nominal)
  Fault Inject/1(Out1) -> Out/1
  StuckAt/1 -> Fault Inject/6(stuckAt)

== system VirtualADAPT/VirtualADAPTv1/EY273_CLFaultInject ==
  In : Inport
  Fault Inject : Subsystem
  Fault Profile : Step  Time=FT  Before=1  After=FP+(FP-1)*3  SampleTime=0
  Filler : Constant  Value=0
  StuckAt : Constant  Value=FM
  Out : Outport
  wires:
  Fault Profile/1 -> Fault Inject/2(Fault Profile)
  Filler/1 -> Fault Inject/3(Step), Fault Inject/4(Ramp), Fault Inject/5(Bias)
  In/1 -> Fault Inject/1(Nominal)
  Fault Inject/1(Out1) -> Out/1
  StuckAt/1 -> Fault Inject/6(stuckAt)

== system VirtualADAPT/VirtualADAPTv1/EY274_CLFaultInject ==
  In : Inport
  Fault Inject : Subsystem
  Fault Profile : Step  Time=FT  Before=1  After=FP+(FP-1)*3  SampleTime=0
  Filler : Constant  Value=0
  StuckAt : Constant  Value=FM
  Out : Outport
  wires:
  Fault Profile/1 -> Fault Inject/2(Fault Profile)
  Filler/1 -> Fault Inject/3(Step), Fault Inject/4(Ramp), Fault Inject/5(Bias)
  In/1 -> Fault Inject/1(Nominal)
  Fault Inject/1(Out1) -> Out/1
  StuckAt/1 -> Fault Inject/6(stuckAt)

== system VirtualADAPT/VirtualADAPTv1/EY275_CLFaultInject ==
  In : Inport
  Fault Inject : Subsystem
  Fault Profile : Step  Time=FT  Before=1  After=FP+(FP-1)*3  SampleTime=0
  Filler : Constant  Value=0
  StuckAt : Constant  Value=FM
  Out : Outport
  wires:
  Fault Profile/1 -> Fault Inject/2(Fault Profile)
  Filler/1 -> Fault Inject/3(Step), Fault Inject/4(Ramp), Fault Inject/5(Bias)
  In/1 -> Fault Inject/1(Nominal)
  Fault Inject/1(Out1) -> Out/1
  StuckAt/1 -> Fault Inject/6(stuckAt)

== system VirtualADAPT/VirtualADAPTv1/EY283_CLFaultInject ==
  In : Inport
  Fault Inject : Subsystem
  Fault Profile : Step  Time=FT  Before=1  After=FP+(FP-1)*3  SampleTime=0
  Filler : Constant  Value=0
  StuckAt : Constant  Value=FM
  Out : Outport
  wires:
  Fault Profile/1 -> Fault Inject/2(Fault Profile)
  Filler/1 -> Fault Inject/3(Step), Fault Inject/4(Ramp), Fault Inject/5(Bias)
  In/1 -> Fault Inject/1(Nominal)
  Fault Inject/1(Out1) -> Out/1
  StuckAt/1 -> Fault Inject/6(stuckAt)

== system VirtualADAPT/VirtualADAPTv1/EY284_CLFaultInject ==
  In : Inport
  Fault Inject : Subsystem
  Fault Profile : Step  Time=FT  Before=1  After=FP+(FP-1)*3  SampleTime=0
  Filler : Constant  Value=0
  StuckAt : Constant  Value=FM
  Out : Outport
  wires:
  Fault Profile/1 -> Fault Inject/2(Fault Profile)
  Filler/1 -> Fault Inject/3(Step), Fault Inject/4(Ramp), Fault Inject/5(Bias)
  In/1 -> Fault Inject/1(Nominal)
  Fault Inject/1(Out1) -> Out/1
  StuckAt/1 -> Fault Inject/6(stuckAt)

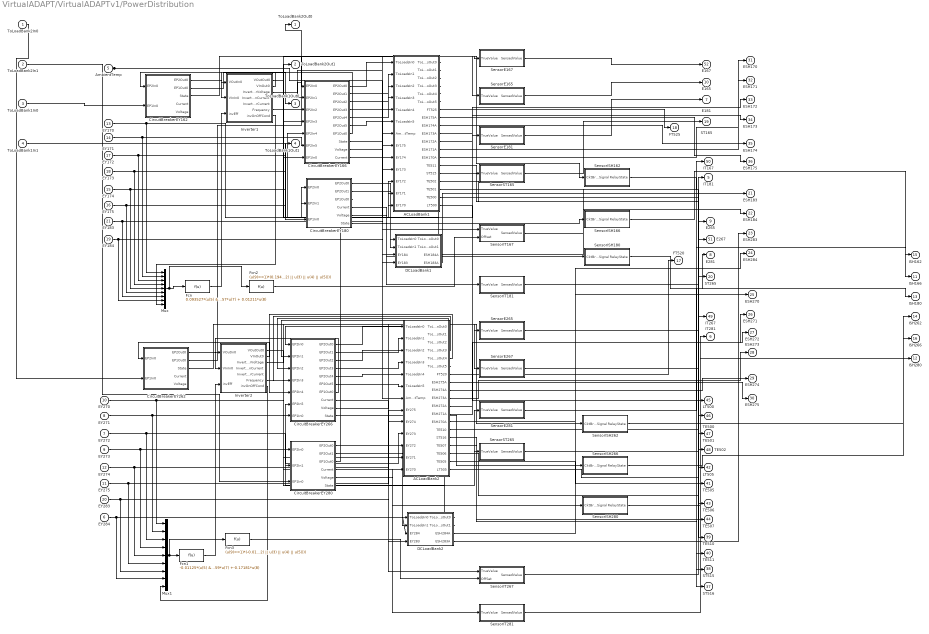
== system VirtualADAPT/VirtualADAPTv1/PowerDistribution ==
  ToLoadBank2In0 : Inport
  ToLoadBank2In1 : Inport  Port=2
  ToLoadBank1In0 : Inport  Port=3
  ToLoadBank1In1 : Inport  Port=4
  AmbientTemp : Inport  Port=5
  EY284 : Inport  Port=6
  EY272 : Inport  Port=7
  EY271 : Inport  Port=8
  EY273 : Inport  Port=9
  EY270 : Inport  Port=10
  EY275 : Inport  Port=11
  EY274 : Inport  Port=12
  EY170 : Inport  Port=13
  EY171 : Inport  Port=14
  EY174 : Inport  Port=15
  EY175 : Inport  Port=16
  EY172 : Inport  Port=17
  EY173 : Inport  Port=18
  EY184 : Inport  Port=19
  EY283 : Inport  Port=20
  EY183 : Inport  Port=21
  ACLoadBank1 : Subsystem
  ACLoadBank2 : Subsystem
  CircuitBreakerEY162 : Subsystem
  CircuitBreakerEY166 : Subsystem
  CircuitBreakerEY180 : Subsystem
  CircuitBreakerEY262 : Subsystem
  CircuitBreakerEY266 : Subsystem
  CircuitBreakerEY280 : Subsystem
  DCLoadBank1 : Subsystem
  DCLoadBank2 : Subsystem
  Fcn : Fcn  Expr=0.093527*(u(5) && (u(1) || u(2) || u(3) || u(4) || u(6))) + 0.059854*(u(3) && (u(1) || u(2) || u(4) || u(5) || u(6))) + 0.0094035*(u(2) && (u(1) || u(3) || u(4) || u(5) || u(6))) + 0.071687*(u(1) && (u(2) || u(3) || u(4) || u(5) || u(6))) + 0.034123*(u(4) && (u(1) || u(2) || u(3) || u(5) || u(6))) + 0.019795*(u(6) && (u(1) || u(2) || u(3) || u(4) || u(5))) + -0.035457*u(7) + 0.01211*u(8)
  Fcn1 : Fcn  Expr=-0.01125*(u(5) && (u(1) || u(2) || u(3) || u(4) || u(6))) + 0.015109*(u(3) && (u(1) || u(2) || u(4) || u(5) || u(6))) + 0.048126*(u(2) && (u(1) || u(3) || u(4) || u(5) || u(6))) + 0.11902*(u(1) && (u(2) || u(3) || u(4) || u(5) || u(6))) + 0.021986*(u(4) && (u(1) || u(2) || u(3) || u(5) || u(6))) + 0.0075553*(u(6) && (u(1) || u(2) || u(3) || u(4) || u(5))) + 0.095759*u(7) +-0.17181*u(8)
  Fcn2 : Fcn  Expr=(u(9)==1)*(0.19498*(u(5) && (u(1) || u(2) || u(3) || u(4) || u(6))) + 0.038661*(u(3) && (u(1) || u(2) || u(4) || u(5) || u(6))) + 0.041019*(u(2) && (u(1) || u(3) || u(4) || u(5) || u(6))) + 0.020674*(u(1) && (u(2) || u(3) || u(4) || u(5) || u(6))) + 0.078975*(u(4) && (u(1) || u(2) || u(3) || u(5) || u(6))) + 0.068196*(u(6) && (u(1) || u(2) || u(3) || u(4) || u(5))))
  Fcn3 : Fcn  Expr=(u(9)==1)*(-0.017764*(u(5) && (u(1) || u(2) || u(3) || u(4) || u(6))) + -0.03402*(u(3) && (u(1) || u(2) || u(4) || u(5) || u(6))) + -0.0064471*(u(2) && (u(1) || u(3) || u(4) || u(5) || u(6))) + 0.19518*(u(1) && (u(2) || u(3) || u(4) || u(5) || u(6))) + 0.052566*(u(4) && (u(1) || u(2) || u(3) || u(5) || u(6))) + 0.044324*(u(6) && (u(1) || u(2) || u(3) || u(4) || u(5))))
  Inverter1 : Subsystem
  Inverter2 : Subsystem
  Mux : Mux  Inputs=9
  Mux1 : Mux  Inputs=9
  SensorE165 : Subsystem
  SensorE167 : Subsystem
  SensorE181 : Subsystem
  SensorE265 : Subsystem
  SensorE267 : Subsystem
  SensorE281 : Subsystem
  SensorISH162 : Subsystem
  SensorISH166 : Subsystem
  SensorISH180 : Subsystem
  SensorISH262 : Subsystem
  SensorISH266 : Subsystem
  SensorISH280 : Subsystem
  SensorIT167 : Subsystem
  SensorIT181 : Subsystem
  SensorIT267 : Subsystem
  SensorIT281 : Subsystem
  SensorST165 : Subsystem
  SensorST265 : Subsystem
  ToLoadBank2Out0 : Outport
  ToLoadBank2Out1 : Outport  Port=2
  ToLoadBank1Out0 : Outport  Port=3
  ToLoadBank1Out1 : Outport  Port=4
  IT181 : Outport  Port=5
  IT281 : Outport  Port=6
  E181 : Outport  Port=7
  E281 : Outport  Port=8
  E265 : Outport  Port=9
  E165 : Outport  Port=10
  ISH166 : Outport  Port=11
  ISH280 : Outport  Port=12
  ISH180 : Outport  Port=13
  ISH262 : Outport  Port=14
  ISH162 : Outport  Port=15
  ISH266 : Outport  Port=16
  FT520 : Outport  Port=17
  FT525 : Outport  Port=18
  ST165 : Outport  Port=19
  ST265 : Outport  Port=20
  ESH183 : Outport  Port=21
  ESH184 : Outport  Port=22
  ESH283 : Outport  Port=23
  ESH284 : Outport  Port=24
  ESH270 : Outport  Port=25
  ESH271 : Outport  Port=26
  ESH272 : Outport  Port=27
  ESH273 : Outport  Port=28
  ESH274 : Outport  Port=29
  ESH275 : Outport  Port=30
  ESH170 : Outport  Port=31
  ESH171 : Outport  Port=32
  ESH172 : Outport  Port=33
  ESH173 : Outport  Port=34
  ESH174 : Outport  Port=35
  ESH175 : Outport  Port=36
  ST516 : Outport  Port=37
  ST515 : Outport  Port=38
  TE510 : Outport  Port=39
  TE511 : Outport  Port=40
  TE505 : Outport  Port=41
  LT505 : Outport  Port=42
  TE506 : Outport  Port=43
  TE507 : Outport  Port=44
  LT500 : Outport  Port=45
  TE500 : Outport  Port=46
  TE501 : Outport  Port=47
  TE502 : Outport  Port=48
  IT267 : Outport  Port=49
  IT167 : Outport  Port=50
  E267 : Outport  Port=51
  E167 : Outport  Port=52
  wires:
  ToLoadBank1In0/1 -> CircuitBreakerEY162/2(EP1In0)
  CircuitBreakerEY162/2(EP1Out0) -> ToLoadBank1Out0/1
  ToLoadBank2In0/1 -> CircuitBreakerEY262/2(EP1In0)
  CircuitBreakerEY262/2(EP1Out0) -> ToLoadBank2Out0/1
  CircuitBreakerEY166/1(EP2Out0) -> ACLoadBank1/1(ToLoadsIn0)
  ACLoadBank1/1(ToLoadsOut0) -> CircuitBreakerEY166/1(EP2In0)
  CircuitBreakerEY166/2(EP2Out1) -> ACLoadBank1/2(ToLoadsIn1)
  ACLoadBank1/2(ToLoadsOut1) -> CircuitBreakerEY166/2(EP2In1)
  CircuitBreakerEY166/3(EP2Out2) -> ACLoadBank1/3(ToLoadsIn2)
  ACLoadBank1/3(ToLoadsOut2) -> CircuitBreakerEY166/3(EP2In2)
  CircuitBreakerEY166/4(EP2Out3) -> ACLoadBank1/4(ToLoadsIn3)
  ACLoadBank1/4(ToLoadsOut3) -> CircuitBreakerEY166/4(EP2In3)
  CircuitBreakerEY166/5(EP2Out4) -> ACLoadBank1/5(ToLoadsIn4)
  ACLoadBank1/5(ToLoadsOut4) -> CircuitBreakerEY166/5(EP2In4)
  CircuitBreakerEY166/6(EP2Out5) -> ACLoadBank1/6(ToLoadsIn5)
  ACLoadBank1/6(ToLoadsOut5) -> CircuitBreakerEY166/6(EP2In5)
  CircuitBreakerEY266/1(EP2Out0) -> ACLoadBank2/1(ToLoadsIn0)
  ACLoadBank2/1(ToLoadsOut0) -> CircuitBreakerEY266/1(EP2In0)
  CircuitBreakerEY266/2(EP2Out1) -> ACLoadBank2/2(ToLoadsIn1)
  ACLoadBank2/2(ToLoadsOut1) -> CircuitBreakerEY266/2(EP2In1)
  CircuitBreakerEY266/3(EP2Out2) -> ACLoadBank2/3(ToLoadsIn2)
  ACLoadBank2/3(ToLoadsOut2) -> CircuitBreakerEY266/3(EP2In2)
  CircuitBreakerEY266/4(EP2Out3) -> ACLoadBank2/4(ToLoadsIn3)
  ACLoadBank2/4(ToLoadsOut3) -> CircuitBreakerEY266/4(EP2In3)
  CircuitBreakerEY266/5(EP2Out4) -> ACLoadBank2/5(ToLoadsIn4)
  ACLoadBank2/5(ToLoadsOut4) -> CircuitBreakerEY266/5(EP2In4)
  CircuitBreakerEY266/6(EP2Out5) -> ACLoadBank2/6(ToLoadsIn5)
  ACLoadBank2/6(ToLoadsOut5) -> CircuitBreakerEY266/6(EP2In5)
  CircuitBreakerEY280/1(EP2Out0) -> DCLoadBank2/1(ToLoadsIn0)
  DCLoadBank2/1(ToLoadsOut0) -> CircuitBreakerEY280/1(EP2In0)
  CircuitBreakerEY280/2(EP2Out1) -> DCLoadBank2/2(ToLoadsIn1)
  DCLoadBank2/2(ToLoadsOut1) -> CircuitBreakerEY280/2(EP2In1)
  ToLoadBank2In1/1 -> CircuitBreakerEY280/3(EP1In0)
  CircuitBreakerEY280/3(EP1Out0) -> ToLoadBank2Out1/1
  CircuitBreakerEY180/1(EP2Out0) -> DCLoadBank1/1(ToLoadsIn0)
  DCLoadBank1/1(ToLoadsOut0) -> CircuitBreakerEY180/1(EP2In0)
  CircuitBreakerEY180/2(EP2Out1) -> DCLoadBank1/2(ToLoadsIn1)
  DCLoadBank1/2(ToLoadsOut1) -> CircuitBreakerEY180/2(EP2In1)
  ToLoadBank1In1/1 -> CircuitBreakerEY180/3(EP1In0)
  CircuitBreakerEY180/3(EP1Out0) -> ToLoadBank1Out1/1
  CircuitBreakerEY162/1(EP2Out0) -> Inverter1/2(VInIn0)
  Inverter1/2(VInOut0) -> CircuitBreakerEY162/1(EP2In0)
  Inverter1/1(VOutOut0) -> CircuitBreakerEY166/7(EP1In0)
  CircuitBreakerEY166/7(EP1Out0) -> Inverter1/1(VOutIn0)
  CircuitBreakerEY262/1(EP2Out0) -> Inverter2/2(VInIn0)
  Inverter2/2(VInOut0) -> CircuitBreakerEY262/1(EP2In0)
  Inverter2/1(VOutOut0) -> CircuitBreakerEY266/7(EP1In0)
  CircuitBreakerEY266/7(EP1Out0) -> Inverter2/1(VOutIn0)
  CircuitBreakerEY162/3(State) -> SensorISH162/1(CktBrkOpenSignal)
  CircuitBreakerEY262/3(State) -> SensorISH262/1(CktBrkOpenSignal)
  CircuitBreakerEY266/8(Current) -> SensorIT267/1(TrueValue)
  CircuitBreakerEY266/9(Voltage) -> SensorE267/1(TrueValue)
  CircuitBreakerEY266/10(State) -> SensorISH266/1(CktBrkOpenSignal)
  CircuitBreakerEY166/8(State) -> SensorISH166/1(CktBrkOpenSignal)
  CircuitBreakerEY166/9(Voltage) -> SensorE167/1(TrueValue)
  CircuitBreakerEY166/10(Current) -> SensorIT167/1(TrueValue)
  CircuitBreakerEY280/4(Current) -> SensorIT281/1(TrueValue)
  CircuitBreakerEY180/4(Current) -> SensorIT181/1(TrueValue)
  SensorIT181/1(SensedValue) -> IT181/1
  SensorIT281/1(SensedValue) -> IT281/1
  SensorE181/1(SensedValue) -> E181/1
  CircuitBreakerEY180/5(Voltage) -> SensorE181/1(TrueValue)
  SensorE281/1(SensedValue) -> E281/1
  CircuitBreakerEY280/5(Voltage) -> SensorE281/1(TrueValue)
  SensorE265/1(SensedValue) -> E265/1
  SensorE165/1(SensedValue) -> E165/1
  CircuitBreakerEY280/6(State) -> SensorISH280/1(CktBrkOpenSignal)
  CircuitBreakerEY180/6(State) -> SensorISH180/1(CktBrkOpenSignal)
  SensorISH280/1(RelayState) -> ISH280/1
  SensorISH266/1(RelayState) -> ISH266/1
  SensorISH262/1(RelayState) -> ISH262/1
  SensorISH180/1(RelayState) -> ISH180/1
  SensorISH166/1(RelayState) -> ISH166/1
  SensorISH162/1(RelayState) -> ISH162/1
  ACLoadBank2/7(FT520) -> FT520/1
  ACLoadBank1/7(FT525) -> FT525/1
  SensorST165/1(SensedValue) -> ST165/1
  SensorST265/1(SensedValue) -> ST265/1
  AmbientTemp/1 -> ACLoadBank2/7(AmbientTemp), ACLoadBank1/7(AmbientTemp)
  SensorIT267/1(SensedValue) -> IT267/1
  SensorIT167/1(SensedValue) -> IT167/1
  ACLoadBank2/8(ESH275A) -> ESH275/1
  ACLoadBank2/9(ESH274A) -> ESH274/1
  ACLoadBank2/10(ESH273A) -> ESH273/1
  ACLoadBank2/11(ESH272A) -> ESH272/1
  ACLoadBank2/12(ESH271A) -> ESH271/1
  ACLoadBank2/13(ESH270A) -> ESH270/1
  ACLoadBank1/8(ESH175A) -> ESH175/1
  ACLoadBank1/9(ESH174A) -> ESH174/1
  ACLoadBank1/10(ESH173A) -> ESH173/1
  ACLoadBank1/11(ESH172A) -> ESH172/1
  ACLoadBank1/12(ESH171A) -> ESH171/1
  ACLoadBank1/13(ESH170A) -> ESH170/1
  DCLoadBank2/3(ESH284A) -> ESH284/1
  DCLoadBank2/4(ESH283A) -> ESH283/1
  DCLoadBank1/3(ESH184A) -> ESH184/1
  DCLoadBank1/4(ESH183A) -> ESH183/1
  ACLoadBank2/14(TE510) -> TE510/1
  ACLoadBank1/14(TE511) -> TE511/1
  ACLoadBank2/15(ST516) -> ST516/1
  ACLoadBank1/15(ST515) -> ST515/1
  ACLoadBank2/16(TE507) -> TE507/1
  ACLoadBank2/17(TE506) -> TE506/1
  ACLoadBank2/18(TE505) -> TE505/1
  ACLoadBank2/19(LT505) -> LT505/1
  ACLoadBank1/16(TE502) -> TE502/1
  ACLoadBank1/17(TE501) -> TE501/1
  ACLoadBank1/18(TE500) -> TE500/1
  ACLoadBank1/19(LT500) -> LT500/1
  EY284/1 -> DCLoadBank2/3(EY284), Mux1/8
  EY275/1 -> ACLoadBank2/8(EY275), Mux1/6
  EY274/1 -> ACLoadBank2/9(EY274), Mux1/5
  EY273/1 -> ACLoadBank2/10(EY273), Mux1/4
  EY272/1 -> ACLoadBank2/11(EY272), Mux1/3
  EY271/1 -> ACLoadBank2/12(EY271), Mux1/2
  EY270/1 -> ACLoadBank2/13(EY270), Mux1/1
  EY175/1 -> ACLoadBank1/8(EY175), Mux/6
  EY174/1 -> ACLoadBank1/9(EY174), Mux/5
  EY173/1 -> ACLoadBank1/10(EY173), Mux/4
  EY172/1 -> ACLoadBank1/11(EY172), Mux/3
  EY171/1 -> ACLoadBank1/12(EY171), Mux/2
  EY170/1 -> ACLoadBank1/13(EY170), Mux/1
  SensorE267/1(SensedValue) -> E267/1
  SensorE167/1(SensedValue) -> E167/1
  EY283/1 -> DCLoadBank2/4(EY283), Mux1/7
  EY184/1 -> DCLoadBank1/3(EY184), Mux/8
  EY183/1 -> DCLoadBank1/4(EY183), Mux/7
  Inverter1/3(InverterVoltage) -> SensorE165/1(TrueValue)
  Inverter1/6(Frequency) -> SensorST165/1(TrueValue)
  Inverter2/3(InverterVoltage) -> SensorE265/1(TrueValue)
  Inverter2/6(Frequency) -> SensorST265/1(TrueValue)
  Mux1/1 -> Fcn1/1, Fcn3/1
  Mux/1 -> Fcn/1, Fcn2/1
  Fcn1/1 -> Inverter2/3(invEff)
  Inverter2/7(invOnOffCond) -> Mux1/9
  Fcn3/1 -> SensorIT267/2(OffSet)
  Fcn2/1 -> SensorIT167/2(Offset)
  Fcn/1 -> Inverter1/3(invEff)
  Inverter1/7(invOnOffCond) -> Mux/9
